simulink model "r_128w_512_11_r370"  (Simulink 7.2) (continued)
== system r_128w_512_11_r370 == (continued)
      mask help: Fold adders into DSPs: Causes adders to be absorbed into DSP blocks (supported in Virtex5)
      mask init: same as r_128w_512_11_r370/pfb_fir_real0
      settings: AttributesFormatString=taps=4, add_latency=1
      mask Help: eval('xlWeb(''http://casper.berkeley.edu/wiki/Pfb_fir_real'')')
      mask StyleString: edit,edit,popup(bartlett|barthannwin|blackman|blackmanharris|bohamwin|chebwin|flattopwin|gausswin|hamming|hann|kaiser|nuttallwin|parzenwin|rectwin|tukeywin|triang|userwindow),edit,edit,edit,edit,edit,edit,edit,edit,edit,edit,popup(Truncate|Round  (unbiased: +/- Inf)|Round  (unbiased: Even Values)),edit,checkbox,edit,checkbox
      mask CallbackString:
      | |%num_taps = eval(get_param(gcb, 'TotalTaps'));
      | %old_vec = eval(get_param(gcb, 'mult_spec'));=-0l
      | 
      | %vec = 2.*ones(1,num_taps);
      | %old_size = length(old_vec);
      | 
      | %if(old_size <= num_taps),
      | %    new_size = old_size;
      | %else,
      | %    new_size = num_taps;
      | %end
      | 
      | %for n = 1:new_size, vec(n) = old_vec(n); end
      | %set_param(gcb, 'mult_spec', mat2str(vec));||||||||||||||%thisVariable = 'specify_mult';
      | %affectedVars = {'mult_spec'};
      | 
      | %thisBlock = gcb();
      | %thisVarState = get_param(thisBlock, thisVariable);
      | %maskNames = get_param(thisBlock, 'MaskNames');
      | %maskEnables = get_param(thisBlock, 'MaskEnables');
      | 
      | %for varName = affectedVars
      | %    varIndex = find(ismember(maskNames, varName));
      | %    maskEnables{varIndex} = thisVarState;
      | %end
      | 
      | %set_param(thisBlock, 'MaskEnables', maskEnables);||
  pfb_fir_real2 : Subsystem  AncestorBlock=casper_library/PFBs/pfb_fir_real  mask(pfb_fir_real){PFBSize=10, TotalTaps=4, WindowType=hamming, n_inputs=2, MakeBiplex=0, BitWidthIn=8, BitWidthOut=18, CoeffBitWidth=12, CoeffDistMem=0, add_latency=1, mult_latency=2, bram_latency=2, conv_latency=2, quantization=Round  (unbiased: Even Values), fwidth=1, specify_mult=off, mult_spec=[2                                      2], adder_folding=on}
      mask prompts: PFBSize "Size of PFB: (2^? pnts)"; TotalTaps "Total Number of Taps:"; WindowType "Windowing Function:"; n_inputs "Number of Simultaneous Inputs: (2^?)"; MakeBiplex "Make Biplex"; BitWidthIn "Input Bitwidth:"; BitWidthOut "Output Bitwidth:"; CoeffBitWidth "Coefficient Bitwidth:"; CoeffDistMem "Use Distributed Memory for Coeffs"; add_latency "Add Latency"; mult_latency "Mult Latency"; bram_latency "BRAM Latency"; conv_latency "Convert latency"; quantization "Quantization Behavior"; fwidth "Bin Width Scaling (normal=1)"; specify_mult "Specify multiplier use (behavioral if not specified)"; mult_spec "Multiplier specification (0=core, 1=embedded, 2=behavioural) (left=1st tap)"; adder_folding "Fold adders into DSPs"
      mask help: Fold adders into DSPs: Causes adders to be absorbed into DSP blocks (supported in Virtex5)
      mask init: same as r_128w_512_11_r370/pfb_fir_real0
      settings: AttributesFormatString=taps=4, add_latency=1
      mask Help: eval('xlWeb(''http://casper.berkeley.edu/wiki/Pfb_fir_real'')')
      mask StyleString: edit,edit,popup(bartlett|barthannwin|blackman|blackmanharris|bohamwin|chebwin|flattopwin|gausswin|hamming|hann|kaiser|nuttallwin|parzenwin|rectwin|tukeywin|triang|userwindow),edit,edit,edit,edit,edit,edit,edit,edit,edit,edit,popup(Truncate|Round  (unbiased: +/- Inf)|Round  (unbiased: Even Values)),edit,checkbox,edit,checkbox
      mask CallbackString:
      | |%num_taps = eval(get_param(gcb, 'TotalTaps'));
      | %old_vec = eval(get_param(gcb, 'mult_spec'));=-0l
      | 
      | %vec = 2.*ones(1,num_taps);
      | %old_size = length(old_vec);
      | 
      | %if(old_size <= num_taps),
      | %    new_size = old_size;
      | %else,
      | %    new_size = num_taps;
      | %end
      | 
      | %for n = 1:new_size, vec(n) = old_vec(n); end
      | %set_param(gcb, 'mult_spec', mat2str(vec));||||||||||||||%thisVariable = 'specify_mult';
      | %affectedVars = {'mult_spec'};
      | 
      | %thisBlock = gcb();
      | %thisVarState = get_param(thisBlock, thisVariable);
      | %maskNames = get_param(thisBlock, 'MaskNames');
      | %maskEnables = get_param(thisBlock, 'MaskEnables');
      | 
      | %for varName = affectedVars
      | %    varIndex = find(ismember(maskNames, varName));
      | %    maskEnables{varIndex} = thisVarState;
      | %end
      | 
      | %set_param(thisBlock, 'MaskEnables', maskEnables);||
  pfb_fir_real3 : Subsystem  AncestorBlock=casper_library/PFBs/pfb_fir_real  mask(pfb_fir_real){PFBSize=10, TotalTaps=4, WindowType=hamming, n_inputs=2, MakeBiplex=0, BitWidthIn=8, BitWidthOut=18, CoeffBitWidth=12, CoeffDistMem=0, add_latency=1, mult_latency=2, bram_latency=2, conv_latency=2, quantization=Round  (unbiased: Even Values), fwidth=1, specify_mult=off, mult_spec=[2                                      2], adder_folding=on}
      mask prompts: PFBSize "Size of PFB: (2^? pnts)"; TotalTaps "Total Number of Taps:"; WindowType "Windowing Function:"; n_inputs "Number of Simultaneous Inputs: (2^?)"; MakeBiplex "Make Biplex"; BitWidthIn "Input Bitwidth:"; BitWidthOut "Output Bitwidth:"; CoeffBitWidth "Coefficient Bitwidth:"; CoeffDistMem "Use Distributed Memory for Coeffs"; add_latency "Add Latency"; mult_latency "Mult Latency"; bram_latency "BRAM Latency"; conv_latency "Convert latency"; quantization "Quantization Behavior"; fwidth "Bin Width Scaling (normal=1)"; specify_mult "Specify multiplier use (behavioral if not specified)"; mult_spec "Multiplier specification (0=core, 1=embedded, 2=behavioural) (left=1st tap)"; adder_folding "Fold adders into DSPs"
      mask help: Fold adders into DSPs: Causes adders to be absorbed into DSP blocks (supported in Virtex5)
      mask init: same as r_128w_512_11_r370/pfb_fir_real0
      settings: AttributesFormatString=taps=4, add_latency=1
      mask Help: eval('xlWeb(''http://casper.berkeley.edu/wiki/Pfb_fir_real'')')
      mask StyleString: edit,edit,popup(bartlett|barthannwin|blackman|blackmanharris|bohamwin|chebwin|flattopwin|gausswin|hamming|hann|kaiser|nuttallwin|parzenwin|rectwin|tukeywin|triang|userwindow),edit,edit,edit,edit,edit,edit,edit,edit,edit,edit,popup(Truncate|Round  (unbiased: +/- Inf)|Round  (unbiased: Even Values)),edit,checkbox,edit,checkbox
      mask CallbackString:
      | |%num_taps = eval(get_param(gcb, 'TotalTaps'));
      | %old_vec = eval(get_param(gcb, 'mult_spec'));=-0l
      | 
      | %vec = 2.*ones(1,num_taps);
      | %old_size = length(old_vec);
      | 
      | %if(old_size <= num_taps),
      | %    new_size = old_size;
      | %else,
      | %    new_size = num_taps;
      | %end
      | 
      | %for n = 1:new_size, vec(n) = old_vec(n); end
      | %set_param(gcb, 'mult_spec', mat2str(vec));||||||||||||||%thisVariable = 'specify_mult';
      | %affectedVars = {'mult_spec'};
      | 
      | %thisBlock = gcb();
      | %thisVarState = get_param(thisBlock, thisVariable);
      | %maskNames = get_param(thisBlock, 'MaskNames');
      | %maskEnables = get_param(thisBlock, 'MaskEnables');
      | 
      | %for varName = affectedVars
      | %    varIndex = find(ismember(maskNames, varName));
      | %    maskEnables{varIndex} = thisVarState;
      | %end
      | 
      | %set_param(thisBlock, 'MaskEnables', maskEnables);||
  pol_pack0 : Subsystem
  pol_pack1 : Subsystem
  pol_pack2 : Subsystem
  pol_pack3 : Subsystem
  posedge{1..2,21,23..24,30} (6 alike) : casper_library/Misc/posedge  Tag=Rising Edge Detector  SystemSampleTime=-1
  pps_count : xps_library/software register  Tag=xps:sw_reg  SystemSampleTime=-1  io_dir=To Processor  arith_type=Unsigned  bitwidth=32  bin_pt=0  sample_period=1  gw_name=r_128w_512_11_r370_pps_count_user_data_in
      link override convert: arith_type=Unsigned  n_bits=32  bin_pt=0
  quant0 : Subsystem  mask{channel_bits=9, din_bit_width=18, din_bin_pt=17, coeff_bit_width=16, coeff_bin_pt=1, dout_bit_width=4, dout_bin_pt=3, init_val=4, overflow=Saturate, quantization=Round  (unbiased: even values), conv_latency=2, add_latency=1, mult_latency=2, bram_latency=2}
      mask prompts: channel_bits "Number channels (2^?)"; din_bit_width "Data input bit width"; din_bin_pt "Data input binary point"; coeff_bit_width "Coefficient bit width"; coeff_bin_pt "Coefficient binary point"; dout_bit_width "Data out bit width"; dout_bin_pt "Data out binary point"; init_val "Initial value"; overflow "Overflow behaviour"; quantization "Quantization Behaviour"; conv_latency "Conversion latency"; add_latency "Adder latency"; mult_latency "Multiplier latency"; bram_latency "BRAM latency"
      mask init:
      | blk = gcb;
      | set_param([blk,'/convert_of0'], ...
      |     'bit_width_i', num2str(din_bit_width+coeff_bit_width+1), ...
      |     'binary_point_i', num2str(din_bin_pt+coeff_bin_pt), ...
      |     'bit_width_o', num2str(dout_bit_width), 'binary_point_o', num2str(dout_bin_pt), ...
      |     'latency', num2str(conv_latency), 'overflow', overflow, ...
      |     'quantization', quantization);
      | set_param([blk,'/convert_of1'], ...
      |     'bit_width_i', num2str(din_bit_width+coeff_bit_width+1), ...
      |     'binary_point_i', num2str(din_bin_pt+coeff_bin_pt), ...
      |     'bit_width_o', num2str(dout_bit_width), 'binary_point_o', num2str(dout_bin_pt), ...
      |     'latency', num2str(conv_latency), 'overflow', overflow, ...
      |     'quantization', quantization);
      | set_param([blk,'/convert_of2'], ...
      |     'bit_width_i', num2str(din_bit_width+coeff_bit_width+1), ...
      |     'binary_point_i', num2str(din_bin_pt+coeff_bin_pt), ...
      |     'bit_width_o', num2str(dout_bit_width), 'binary_point_o', num2str(dout_bin_pt), ...
      |     'latency', num2str(conv_latency), 'overflow', overflow, ...
      |     'quantization', quantization);
      | set_param([blk,'/convert_of3'], ...
      |     'bit_width_i', num2str(din_bit_width+coeff_bit_width+1), ...
      |     'binary_point_i', num2str(din_bin_pt+coeff_bin_pt), ...
      |     'bit_width_o', num2str(dout_bit_width), 'binary_point_o', num2str(dout_bin_pt), ...
      |     'latency', num2str(conv_latency), 'overflow', overflow, ...
      |     'quantization', quantization);
      mask StyleString: edit,edit,edit,edit,edit,edit,edit,edit,popup(Wrap|Saturate|Error),popup(Truncate|Round  (unbiased: +/- Inf)|Round  (unbiased: even values)),edit,edit,edit,edit
  quant1 : Subsystem  mask{channel_bits=9, din_bit_width=18, din_bin_pt=17, coeff_bit_width=16, coeff_bin_pt=1, dout_bit_width=4, dout_bin_pt=3, init_val=4, overflow=Saturate, quantization=Round  (unbiased: even values), conv_latency=2, add_latency=1, mult_latency=2, bram_latency=2}
      mask prompts: channel_bits "Number channels (2^?)"; din_bit_width "Data input bit width"; din_bin_pt "Data input binary point"; coeff_bit_width "Coefficient bit width"; coeff_bin_pt "Coefficient binary point"; dout_bit_width "Data out bit width"; dout_bin_pt "Data out binary point"; init_val "Initial value"; overflow "Overflow behaviour"; quantization "Quantization Behaviour"; conv_latency "Conversion latency"; add_latency "Adder latency"; mult_latency "Multiplier latency"; bram_latency "BRAM latency"
      mask init: same as r_128w_512_11_r370/quant0
      mask StyleString: edit,edit,edit,edit,edit,edit,edit,edit,popup(Wrap|Saturate|Error),popup(Truncate|Round  (unbiased: +/- Inf)|Round  (unbiased: even values)),edit,edit,edit,edit
  quant2 : Subsystem  mask{channel_bits=9, din_bit_width=18, din_bin_pt=17, coeff_bit_width=16, coeff_bin_pt=1, dout_bit_width=4, dout_bin_pt=3, init_val=4, overflow=Saturate, quantization=Round  (unbiased: even values), conv_latency=2, add_latency=1, mult_latency=2, bram_latency=2}
      mask prompts: channel_bits "Number channels (2^?)"; din_bit_width "Data input bit width"; din_bin_pt "Data input binary point"; coeff_bit_width "Coefficient bit width"; coeff_bin_pt "Coefficient binary point"; dout_bit_width "Data out bit width"; dout_bin_pt "Data out binary point"; init_val "Initial value"; overflow "Overflow behaviour"; quantization "Quantization Behaviour"; conv_latency "Conversion latency"; add_latency "Adder latency"; mult_latency "Multiplier latency"; bram_latency "BRAM latency"
      mask init: same as r_128w_512_11_r370/quant0
      mask StyleString: edit,edit,edit,edit,edit,edit,edit,edit,popup(Wrap|Saturate|Error),popup(Truncate|Round  (unbiased: +/- Inf)|Round  (unbiased: even values)),edit,edit,edit,edit
  quant3 : Subsystem  mask{channel_bits=9, din_bit_width=18, din_bin_pt=17, coeff_bit_width=16, coeff_bin_pt=1, dout_bit_width=4, dout_bin_pt=3, init_val=4, overflow=Saturate, quantization=Round  (unbiased: even values), conv_latency=2, add_latency=1, mult_latency=2, bram_latency=2}
      mask prompts: channel_bits "Number channels (2^?)"; din_bit_width "Data input bit width"; din_bin_pt "Data input binary point"; coeff_bit_width "Coefficient bit width"; coeff_bin_pt "Coefficient binary point"; dout_bit_width "Data out bit width"; dout_bin_pt "Data out binary point"; init_val "Initial value"; overflow "Overflow behaviour"; quantization "Quantization Behaviour"; conv_latency "Conversion latency"; add_latency "Adder latency"; mult_latency "Multiplier latency"; bram_latency "BRAM latency"
      mask init: same as r_128w_512_11_r370/quant0
      mask StyleString: edit,edit,edit,edit,edit,edit,edit,edit,popup(Wrap|Saturate|Error),popup(Truncate|Round  (unbiased: +/- Inf)|Round  (unbiased: even values)),edit,edit,edit,edit
  quant_snap0 : casper_library/Scopes/snap  SystemSampleTime=-1  nsamples=10
  repack0 : Subsystem
  revision_control : Subsystem  AncestorBlock=casper_library/Misc/revision_control  mask(revision_control){rev_major=0, rev_minor=370, error_on_dirty=off, error_on_norcs=off}
      mask prompts: rev_major "Major revision number"; rev_minor "Minor revision number"; error_on_dirty "Error on out-of-date"; error_on_norcs "Error on no revision control"
      mask init:
      | revision_control_init( gcb, ...
      |   'rev_major', rev_major, ...
      |   'rev_minor', rev_minor, ...
      |   'error_on_dirty', error_on_dirty, ...
      |   'error_on_norcs', error_on_norcs);
  timing : Subsystem  mask{reset_period=1, sync_gen_bits=27}
      mask prompts: reset_period "Master reset time (sync pulses)"; sync_gen_bits "Size of sync generator counter (bits)"
  unpack0 : Subsystem
  unpack1 : Subsystem
  unpack2 : Subsystem
  unpack3 : Subsystem
  wires:
  [adc_sync0] -> Logical3/2
  [adc0] -> Concat/1
  [adc1] -> Concat/2
  timing/2(sync) -> [sync]
  timing/3(data_out) -> adc_unpack/1(In1)
  adc_unpack/2(Out2) -> [data_adc1]
  Logical3/1 -> timing/4(pps), [pps]
  Concat/1 -> timing/2(data_in)
  Constant9/1 -> fft_shift0/1
  fft_shift0/1 -> Slice6/1
  a0p0_1st_shift/2(dout1) -> Gateway Out1/1, fft_wideband_real0/3(in0)
  a0p0_1st_shift/3(dout2) -> Gateway Out2/1, fft_wideband_real0/4(in1)
  a0p0_1st_shift/4(dout3) -> Gateway Out3/1, fft_wideband_real0/5(in2)
  a0p0_1st_shift/5(dout4) -> Gateway Out4/1, fft_wideband_real0/6(in3)
  Slice6/1 -> Gateway Out5/1, fft_wideband_real0/2(shift)
  a0p0_1st_shift/1(sync_out) -> Gateway Out/1, fft_wideband_real0/1(sync)
  fft_wideband_real0/1(sync_out) -> Gateway Out6/1, [fft_sync], fd_fs0/1(sync)
  fft_wideband_real0/2(out0) -> c_to_ri6/1, fd_fs0/8(din0)
  fft_wideband_real0/3(out1) -> c_to_ri7/1, fd_fs0/9(din1)
  fft_wideband_real0/4(of) -> [fft_or0]
  Gateway Out/1 -> Scope/1
  Gateway Out1/1 -> Scope/2
  Gateway Out2/1 -> Scope/3
  Gateway Out3/1 -> Scope/4
  Gateway Out4/1 -> Scope/5
  Gateway Out5/1 -> Scope/6
  adc_unpack/1(Out1) -> [data_adc0]
  [adc2] -> Concat/3
  [adc3] -> Concat/4
  adc_unpack/3(Out3) -> [data_adc2]
  adc_unpack/4(Out4) -> [data_adc3]
  pfb_fir_real1/2(pol1_out1) -> a0p1_1st_shift/2(din1)
  pfb_fir_real1/3(pol1_out2) -> a0p1_1st_shift/3(din2)
  pfb_fir_real1/4(pol1_out3) -> a0p1_1st_shift/4(din3)
  pfb_fir_real1/5(pol1_out4) -> a0p1_1st_shift/5(din4)
  a0p1_1st_shift/2(dout1) -> fft_wideband_real1/3(in0)
  a0p1_1st_shift/3(dout2) -> fft_wideband_real1/4(in1)
  a0p1_1st_shift/4(dout3) -> fft_wideband_real1/5(in2)
  a0p1_1st_shift/5(dout4) -> fft_wideband_real1/6(in3)
  pfb_fir_real1/1(sync_out) -> a0p1_1st_shift/1(sync_in)
  a0p1_1st_shift/1(sync_out) -> fft_wideband_real1/1(sync)
  fft_wideband_real1/4(of) -> [fft_or1]
  delay_wideband_prog2/1(sync_out) -> pfb_fir_real2/1(sync)
  delay_wideband_prog2/2(data_out1) -> pfb_fir_real2/2(pol1_in1)
  delay_wideband_prog2/3(data_out2) -> pfb_fir_real2/3(pol1_in2)
  delay_wideband_prog2/4(data_out3) -> pfb_fir_real2/4(pol1_in3)
  delay_wideband_prog2/5(data_out4) -> pfb_fir_real2/5(pol1_in4)
  pfb_fir_real2/2(pol1_out1) -> a1p0_1st_shift/2(din1)
  pfb_fir_real2/3(pol1_out2) -> a1p0_1st_shift/3(din2)
  pfb_fir_real2/4(pol1_out3) -> a1p0_1st_shift/4(din3)
  pfb_fir_real2/5(pol1_out4) -> a1p0_1st_shift/5(din4)
  a1p0_1st_shift/2(dout1) -> fft_wideband_real2/3(in0)
  a1p0_1st_shift/3(dout2) -> fft_wideband_real2/4(in1)
  a1p0_1st_shift/4(dout3) -> fft_wideband_real2/5(in2)
  a1p0_1st_shift/5(dout4) -> fft_wideband_real2/6(in3)
  pfb_fir_real2/1(sync_out) -> a1p0_1st_shift/1(sync_in)
  a1p0_1st_shift/1(sync_out) -> fft_wideband_real2/1(sync)
  fft_wideband_real2/4(of) -> [fft_or2]
  Concat5/1 -> [quant2_data]
  delay_wideband_prog3/1(sync_out) -> pfb_fir_real3/1(sync)
  delay_wideband_prog3/2(data_out1) -> pfb_fir_real3/2(pol1_in1)
  delay_wideband_prog3/3(data_out2) -> pfb_fir_real3/3(pol1_in2)
  delay_wideband_prog3/4(data_out3) -> pfb_fir_real3/4(pol1_in3)
  delay_wideband_prog3/5(data_out4) -> pfb_fir_real3/5(pol1_in4)
  pfb_fir_real3/2(pol1_out1) -> a1p1_1st_shift/2(din1)
  pfb_fir_real3/3(pol1_out2) -> a1p1_1st_shift/3(din2)
  pfb_fir_real3/4(pol1_out3) -> a1p1_1st_shift/4(din3)
  pfb_fir_real3/5(pol1_out4) -> a1p1_1st_shift/5(din4)
  a1p1_1st_shift/2(dout1) -> fft_wideband_real3/3(in0)
  a1p1_1st_shift/3(dout2) -> fft_wideband_real3/4(in1)
  a1p1_1st_shift/4(dout3) -> fft_wideband_real3/5(in2)
  a1p1_1st_shift/5(dout4) -> fft_wideband_real3/6(in3)
  pfb_fir_real3/1(sync_out) -> a1p1_1st_shift/1(sync_in)
  a1p1_1st_shift/1(sync_out) -> fft_wideband_real3/1(sync)
  fft_wideband_real3/4(of) -> [fft_or3]
  quant3/2(dout0) -> Subsystem1/5(In5), Concat8/1
  quant3/3(dout1) -> Concat8/2
  Concat8/1 -> [quant3_data]
  quant3/4(of) -> Subsystem1/9(In9), [quant_or3]
  Gateway Out6/1 -> Scope2/1
  Gateway Out7/1 -> Scope2/2
  Gateway Out8/1 -> Scope2/3
  Gateway Out9/1 -> Scope2/4
  Gateway Out10/1 -> Scope2/5
  c_to_ri6/1 -> Gateway Out7/1
  c_to_ri6/2 -> Gateway Out8/1
  c_to_ri7/1 -> Gateway Out9/1
  c_to_ri7/2 -> Gateway Out10/1
  unpack0/1(Out1) -> Gateway Out12/1, delay_wideband_prog0/4(data_in1)
  unpack0/2(Out2) -> Gateway Out13/1, delay_wideband_prog0/5(data_in2)
  unpack0/3(Out3) -> Gateway Out14/1, delay_wideband_prog0/6(data_in3)
  unpack0/4(Out4) -> Gateway Out15/1, delay_wideband_prog0/7(data_in4)
  delay_wideband_prog1/1(sync_out) -> pfb_fir_real1/1(sync)
  delay_wideband_prog1/2(data_out1) -> pfb_fir_real1/2(pol1_in1)
  delay_wideband_prog1/3(data_out2) -> pfb_fir_real1/3(pol1_in2)
  delay_wideband_prog1/4(data_out3) -> pfb_fir_real1/4(pol1_in3)
  delay_wideband_prog1/5(data_out4) -> pfb_fir_real1/5(pol1_in4)
  delay_wideband_prog0/1(sync_out) -> Gateway Out17/1, pfb_fir_real0/1(sync)
  delay_wideband_prog0/2(data_out1) -> Gateway Out18/1, pfb_fir_real0/2(pol1_in1)
  delay_wideband_prog0/3(data_out2) -> Gateway Out19/1, pfb_fir_real0/3(pol1_in2)
  delay_wideband_prog0/4(data_out3) -> Gateway Out20/1, pfb_fir_real0/4(pol1_in3)
  delay_wideband_prog0/5(data_out4) -> Gateway Out21/1, pfb_fir_real0/5(pol1_in4)
  Gateway Out11/1 -> Scope3/1
  Gateway Out12/1 -> Scope3/2
  Gateway Out13/1 -> Scope3/3
  Gateway Out14/1 -> Scope3/4
  Gateway Out15/1 -> Scope3/5
  Gateway Out16/1 -> Scope3/6
  Gateway Out17/1 -> Scope4/1
  Gateway Out18/1 -> Scope4/2
  Gateway Out19/1 -> Scope4/3
  Gateway Out20/1 -> Scope4/4
  Gateway Out21/1 -> Scope4/5
  Gateway Out22/1 -> Scope5/1
  Gateway Out23/1 -> Scope5/2
  Gateway Out24/1 -> Scope5/3
  Gateway Out25/1 -> Scope5/4
  Gateway Out26/1 -> Scope5/5
  [adc_sync1] -> Logical3/3
  quant2/2(dout0) -> Subsystem1/4(In4), Concat5/1
  quant2/3(dout1) -> Concat5/2
  quant2/4(of) -> Subsystem1/8(In8), [quant_or2]
  quant1/2(dout0) -> Subsystem1/3(In3), Concat3/1
  quant1/3(dout1) -> Concat3/2
  Concat3/1 -> [quant1_data]
  quant1/4(of) -> Subsystem1/7(In7), [quant_or1]
  quant0/2(dout0) -> Concat7/1, Subsystem1/2(In2)
  quant0/3(dout1) -> Concat7/2
  quant0/1(sync_out) -> Subsystem1/1(In1), [quant0_sync]
  quant0/4(of) -> [quant_or0], Subsystem1/6(In6)
  Concat7/1 -> [quant0_data]
  timing/1(armed) -> Logical14/2, [armed]
  Logical14/1 -> [mrst]
  [mrst] -> cd_local_time/1(reset)
  [sync] -> cd_local_time/2(sync)
  cd_local_time/1(time) -> [cd_local_time]
  [sys_rst] -> Logical14/1
  [man_sync] -> Logical3/1
  [arm] -> timing/1(arm)
  Constant2/1 -> timing/3(sync_period)
  pfb_fir_real0/2(pol1_out1) -> a0p0_1st_shift/2(din1), Gateway Out23/1
  pfb_fir_real0/3(pol1_out2) -> a0p0_1st_shift/3(din2), Gateway Out24/1
  pfb_fir_real0/4(pol1_out3) -> a0p0_1st_shift/4(din3), Gateway Out25/1
  pfb_fir_real0/5(pol1_out4) -> a0p0_1st_shift/5(din4), Gateway Out26/1
  pfb_fir_real0/1(sync_out) -> a0p0_1st_shift/1(sync_in), Gateway Out22/1
  quant1/1(sync_out) -> Terminator6/1
  quant2/1(sync_out) -> Terminator7/1
  quant3/1(sync_out) -> Terminator8/1
  Constant33/1 -> ld_time_msw0/1
  Constant29/1 -> ld_time_lsw0/1
  ld_time_msw0/1 -> Slice73/1, Slice7/1
  Slice73/1 -> Concat9/1
  ld_time_lsw0/1 -> Concat9/2
  fstatus0/1 -> Terminator9/1
  Constant18/1 -> control/1
  control/1 -> Delay6/1
  Subsystem/1(status) -> fstatus0/1
  Delay6/1 -> Slice78/1, man_sync1/1, arm1/1, arm2/1, arm3/1, arm4/1, arm5/1, arm6/1, arm7/1
  Slice78/1 -> [sys_rst]
  man_sync1/1 -> posedge1/1
  posedge1/1 -> [man_sync]
  arm1/1 -> posedge24/1
  posedge24/1 -> [arm]
  arm2/1 -> posedge23/1
  posedge23/1 -> [clr_status]
  Counter1/1 -> Register19/1
  Register19/1 -> clk_frequency/1
  [pps] -> Counter1/1, Counter3/2, Register19/2
  clk_frequency/1 -> Terminator10/1
  pps_count/1 -> Terminator11/1
  [mrst] -> Counter3/1
  [cd_local_time] -> Delay7/1
  mcount_msw/1 -> Terminator12/1
  mcount_lsw/1 -> Terminator13/1
  Slice/1 -> mcount_lsw/1
  Delay7/1 -> Slice1/1, Slice/1
  Slice1/1 -> mcount_msw/1
  Constant1/1 -> adc_snap0/3
  [sync] -> adc_snap0/2
  Constant6/1 -> adc_snap1/3
  [data_adc1] -> adc_snap1/1
  [sync] -> adc_snap1/2
  Constant7/1 -> adc_snap2/3
  [data_adc2] -> adc_snap2/1
  [sync] -> adc_snap2/2
  [data_adc0] -> adc_snap0/1
  Constant19/1 -> adc_snap3/3
  [sync] -> adc_snap3/2
  [data_adc3] -> adc_snap3/1
  Logical7/1 -> Logical11/1
  Logical10/1 -> Logical11/2
  [sync] -> Counter/2
  [mrst] -> Counter/1
  Logical11/1 -> leds/1(warning)
  Counter/1 -> leds/2(sync_led)
  [pps] -> Counter2/1
  Counter2/1 -> leds/3(pps)
  [adc_or0] -> Logical7/1
  [fft_or0] -> Logical7/2
  [adc_or1] -> Logical7/3
  [fft_or1] -> Logical7/4
  [adc_or0] -> Logical10/1
  [fft_or2] -> Logical10/2
  [adc_or1] -> Logical10/3
  [fft_or3] -> Logical10/4
  fstatus1/1 -> Terminator2/1
  fstatus2/1 -> Terminator3/1
  fstatus3/1 -> Terminator4/1
  Constant32/1 -> coarse_delay0/1
  Constant8/1 -> a0_fd0/1
  Constant4/1 -> a1_fd0/1
  a0_fd0/1 -> Slice3/1, Slice2/1
  a1_fd0/1 -> Slice5/1, Slice4/1
  Slice4/1 -> Concat22/4
  Slice5/1 -> Concat22/5
  Concat9/1 -> cd_tr0/4(load_time), fd_tr0/4(load_time)
  [cd_local_time] -> cd_tr0/3(current_time)
  Slice7/1 -> fd_tr0/2(arm), cd_tr0/2(arm)
  [mrst] -> cd_tr0/1(rst)
  delay_tr_status0/1 -> Terminator14/1
  cd_tr0/1(arm_count) -> Convert/1
  cd_tr0/4(ld_count) -> Convert1/1
  cd_tr0/3(enable) -> [cd_en0]
  cd_tr0/2(value) -> [cd_val0]
  Convert/1 -> Concat2/1
  Convert1/1 -> Concat2/2
  Concat2/1 -> delay_tr_status0/1
  fd_tr0/2(value) -> [fd_val0]
  fd_tr0/3(enable) -> [fd_en0]
  delay_tr_status1/1 -> Terminator16/1
  cd_tr1/1(arm_count) -> Convert4/1
  cd_tr1/2(value) -> [cd_val1]
  Convert4/1 -> Concat10/1
  Convert5/1 -> Concat10/2
  Concat10/1 -> delay_tr_status1/1
  fd_tr1/2(value) -> [fd_val1]
  [cd_local_time] -> cd_tr2/3(current_time)
  [mrst] -> fd_tr2/1(rst)
  fd_fs0/3(dout1) -> quant0/7(din1)
  Constant27/1 -> adc_ctrl0/1
  adc_ctrl0/1 -> Slice29/1, Slice30/1
  [eq_real0] -> quant0/1(real_in0)
  Logical/1 -> posedge21/1
  Logical6/1 -> posedge30/1
  posedge21/1 -> [adc_sync0]
  posedge30/1 -> [adc_sync1]
  [mrst] -> fd_local_time/1(reset)
  [fft_sync] -> fd_local_time/2(sync)
  fd_local_time/1(time) -> [fd_local_time]
  Constant43/1 -> XAUI0/1
  Constant44/1 -> XAUI0/2
  packetiser0/1(data_out) -> XAUI0/3
  packetiser0/2(oob) -> XAUI0/4
  packetiser0/3(valid_out) -> XAUI0/5
  XAUI0/2 -> Terminator27/1
  XAUI0/3 -> Terminator32/1
  XAUI0/6 -> [xaui_of0]
  Logical4/1 -> neg_edge_ext4/1(in)
  XAUI0/4 -> Terminator33/1
  XAUI0/1 -> Terminator26/1
  neg_edge_ext4/1(out) -> leds/4(comms)
  [xaui_of0] -> Logical4/1
  [xaui_ldn0] -> Logical4/2
  [xaui_of1] -> Logical4/3
  [xaui_ldn1] -> Logical4/4
  Subsystem2/1(status) -> fstatus1/1
  Subsystem3/1(status) -> fstatus2/1
  Subsystem4/1(status) -> fstatus3/1
  [armed] -> Concat21/1
  Counter3/1 -> Concat21/2
  Concat21/1 -> pps_count/1
  Constant40/1 -> fft_shift1/1
  fft_shift1/1 -> Slice26/1
  Slice26/1 -> fft_wideband_real1/2(shift)
  Constant42/1 -> fft_shift2/1
  fft_shift2/1 -> Slice27/1
  Slice27/1 -> fft_wideband_real2/2(shift)
  Constant45/1 -> fft_shift3/1
  fft_shift3/1 -> Slice28/1
  Slice28/1 -> fft_wideband_real3/2(shift)
  XAUI0/5 -> [xaui_ldn0]
  calc_adc_sum_sq/1(sum_sq) -> adc_sum_sq0/1
  decat0/1(Out1) -> calc_adc_sum_sq/1(In1)
  decat0/2(Out2) -> calc_adc_sum_sq/2(In2)
  decat0/3(Out3) -> calc_adc_sum_sq/3(In3)
  decat0/4(Out4) -> calc_adc_sum_sq/4(In4)
  [adc0] -> decat0/1(In1)
  adc_sum_sq0/1 -> Terminator/1
  [adc1] -> decat1/1(In1)
  [adc2] -> decat2/1(In1)
  [adc3] -> decat3/1(In1)
  katadc0/15(data_valid) -> Terminator1/1
  katadc0/1(i0) -> pol_pack0/1(In1)
  katadc0/2(i1) -> pol_pack0/2(In2)
  katadc0/3(i2) -> pol_pack0/3(In3)
  katadc0/4(i3) -> pol_pack0/4(In4)
  katadc0/5(q0) -> pol_pack1/1(In1)
  katadc0/6(q1) -> pol_pack1/2(In2)
  katadc0/7(q2) -> pol_pack1/3(In3)
  katadc0/8(q3) -> pol_pack1/4(In4)
  katadc0/11(sync0) -> Logical/1
  katadc0/12(sync1) -> Logical/2
  katadc0/13(sync2) -> Logical/3
  katadc0/14(sync3) -> Logical/4
  katadc1/1(i0) -> pol_pack2/1(In1)
  katadc1/2(i1) -> pol_pack2/2(In2)
  katadc1/3(i2) -> pol_pack2/3(In3)
  katadc1/4(i3) -> pol_pack2/4(In4)
  katadc1/5(q0) -> pol_pack3/1(In1)
  katadc1/6(q1) -> pol_pack3/2(In2)
  katadc1/7(q2) -> pol_pack3/3(In3)
  katadc1/8(q3) -> pol_pack3/4(In4)
  katadc1/11(sync0) -> Logical6/1
  katadc1/12(sync1) -> Logical6/2
  katadc1/13(sync2) -> Logical6/3
  katadc1/14(sync3) -> Logical6/4
  katadc1/15(data_valid) -> Terminator5/1
  Constant/1 -> katadc0/8(sim_data_valid)
  Pulse Generator/1 -> katadc0/7(sim_sync)
  Pulse Generator2/1 -> katadc0/6(sim_q)
  Pulse Generator1/1 -> katadc0/3(sim_i)
  Slice29/1 -> katadc0/1(en_i)
  Slice30/1 -> katadc0/2(atten_i)
  Constant48/1 -> adc_ctrl1/1
  adc_ctrl1/1 -> Slice34/1, Slice33/1
  Slice33/1 -> katadc0/4(en_q)
  Slice34/1 -> katadc0/5(atten_q)
  Constant49/1 -> adc_ctrl2/1
  adc_ctrl2/1 -> Slice32/1, Slice31/1
  Constant5/1 -> katadc1/8(sim_data_valid)
  Pulse Generator3/1 -> katadc1/7(sim_sync)
  Pulse Generator5/1 -> katadc1/6(sim_q)
  Pulse Generator4/1 -> katadc1/3(sim_i)
  Slice31/1 -> katadc1/1(en_i)
  Slice32/1 -> katadc1/2(atten_i)
  Constant50/1 -> adc_ctrl3/1
  adc_ctrl3/1 -> Slice35/1, Slice36/1
  Slice35/1 -> katadc1/4(en_q)
  Slice36/1 -> katadc1/5(atten_q)
  calc_adc_sum_sq1/1(sum_sq) -> adc_sum_sq1/1
  decat1/1(Out1) -> calc_adc_sum_sq1/1(In1)
  decat1/2(Out2) -> calc_adc_sum_sq1/2(In2)
  decat1/3(Out3) -> calc_adc_sum_sq1/3(In3)
  decat1/4(Out4) -> calc_adc_sum_sq1/4(In4)
  adc_sum_sq1/1 -> Terminator25/1
  calc_adc_sum_sq2/1(sum_sq) -> adc_sum_sq2/1
  decat2/1(Out1) -> calc_adc_sum_sq2/1(In1)
  decat2/2(Out2) -> calc_adc_sum_sq2/2(In2)
  decat2/3(Out3) -> calc_adc_sum_sq2/3(In3)
  decat2/4(Out4) -> calc_adc_sum_sq2/4(In4)
  adc_sum_sq2/1 -> Terminator30/1
  calc_adc_sum_sq3/1(sum_sq) -> adc_sum_sq3/1
  decat3/1(Out1) -> calc_adc_sum_sq3/1(In1)
  decat3/2(Out2) -> calc_adc_sum_sq3/2(In2)
  decat3/3(Out3) -> calc_adc_sum_sq3/3(In3)
  decat3/4(Out4) -> calc_adc_sum_sq3/4(In4)
  adc_sum_sq3/1 -> Terminator31/1
  Constant3/1 -> board_id/1
  board_id/1 -> [board_id]
  arm3/1 -> posedge2/1
  posedge2/1 -> [tvg_en]
  arm4/1 -> [ct_tvg]
  arm5/1 -> [packetiser_tvg]
  arm6/1 -> [fd_fs_tvg]
  repack0/1(dout0) -> quant_snap0/1
  repack0/2(sync_out) -> quant_snap0/2, Counter4/1
  [quant0_sync] -> repack0/1(sync)
  [quant0_data] -> repack0/2(din0)
  Counter4/1 -> Slice37/1
  Slice37/1 -> quant_snap0/3
  [clr_status] -> Subsystem/1(clr)
  [xaui_ldn0] -> Subsystem/2(xuai_ldn)
  [xaui_of0] -> Subsystem/3(xaui_of)
  [ct_err0] -> Subsystem/4(ct_err)
  [adc_or0] -> Subsystem/5(adc_or)
  [fft_or0] -> Subsystem/6(fft_or)
  [quant_or0] -> Subsystem/7(quant_or)
  [clr_status] -> Subsystem2/1(clr)
  [xaui_ldn0] -> Subsystem2/2(xuai_ldn)
  [xaui_of0] -> Subsystem2/3(xaui_of)
  [ct_err0] -> Subsystem2/4(ct_err)
  [adc_or0] -> Subsystem2/5(adc_or)
  [fft_or1] -> Subsystem2/6(fft_or)
  [quant_or1] -> Subsystem2/7(quant_or)
  [clr_status] -> Subsystem3/1(clr)
  [xaui_ldn1] -> Subsystem3/2(xuai_ldn)
  [xaui_of1] -> Subsystem3/3(xaui_of)
  [ct_err1] -> Subsystem3/4(ct_err)
  [adc_or1] -> Subsystem3/5(adc_or)
  [fft_or2] -> Subsystem3/6(fft_or)
  [quant_or2] -> Subsystem3/7(quant_or)
  [clr_status] -> Subsystem4/1(clr)
  [xaui_ldn1] -> Subsystem4/2(xuai_ldn)
  [xaui_of1] -> Subsystem4/3(xaui_of)
  [ct_err1] -> Subsystem4/4(ct_err)
  [adc_or1] -> Subsystem4/5(adc_or)
  [fft_or3] -> Subsystem4/6(fft_or)
  [quant_or3] -> Subsystem4/7(quant_or)
  katadc0/9(outofrange0) -> Logical1/1
  katadc0/10(outofrange1) -> Logical1/2
  Logical1/1 -> [adc_or0]
  Logical2/1 -> [adc_or1]
  katadc1/9(outofrange0) -> Logical2/1
  katadc1/10(outofrange1) -> Logical2/2
  [mrst] -> packetiser0/4(mrst_in)
  [board_id] -> packetiser0/5(ant_base)
  [quant0_sync] -> ct0/1(sync)
  [quant0_data] -> ct0/2(p0)
  [quant1_data] -> ct0/3(p1)
  ct0/4(ct_err) -> [ct_err0]
  [quant2_data] -> ct1/2(p0)
  fd_fs0/1(sync_out) -> [fd_sync], quant0/5(sync_in)
  [fd_sync] -> Counter8/1
  Counter8/1 -> eq0/1(addr)
  eq0/1(data_out) -> Slice41/1, Slice42/1
  Slice41/1 -> c_to_ri/1
  Slice42/1 -> c_to_ri1/1
  Constant41/1 -> eq0/2(data_in)
  Constant46/1 -> eq0/3(we)
  c_to_ri/1 -> [eq_real0]
  c_to_ri/2 -> [eq_imag0]
  c_to_ri1/1 -> [eq_real1]
  c_to_ri1/2 -> [eq_imag1]
  [fd_sync] -> Counter9/1
  Counter9/1 -> eq1/1(addr)
  eq1/1(data_out) -> Slice44/1, Slice43/1
  Slice43/1 -> c_to_ri2/1
  Slice44/1 -> c_to_ri3/1
  Constant28/1 -> eq1/2(data_in)
  Constant30/1 -> eq1/3(we)
  c_to_ri2/1 -> [eq_real2]
  c_to_ri2/2 -> [eq_imag2]
  c_to_ri3/1 -> [eq_real3]
  c_to_ri3/2 -> [eq_imag3]
  [fd_sync] -> Counter10/1
  Counter10/1 -> eq2/1(addr)
  eq2/1(data_out) -> Slice46/1, Slice45/1
  Slice45/1 -> c_to_ri4/1
  Slice46/1 -> c_to_ri5/1
  Constant35/1 -> eq2/2(data_in)
  Constant36/1 -> eq2/3(we)
  c_to_ri4/1 -> [eq_real4]
  c_to_ri4/2 -> [eq_imag4]
  c_to_ri5/1 -> [eq_real5]
  c_to_ri5/2 -> [eq_imag5]
  [fd_sync] -> Counter11/1
  Counter11/1 -> eq3/1(addr)
  eq3/1(data_out) -> Slice47/1, Slice48/1
  Slice47/1 -> c_to_ri8/1
  Slice48/1 -> c_to_ri9/1
  Constant31/1 -> eq3/2(data_in)
  Constant34/1 -> eq3/3(we)
  c_to_ri8/1 -> [eq_real6]
  c_to_ri8/2 -> [eq_imag6]
  c_to_ri9/1 -> [eq_real7]
  c_to_ri9/2 -> [eq_imag7]
  [eq_imag0] -> quant0/2(imag_in0)
  [eq_real1] -> quant0/3(real_in1)
  [eq_imag1] -> quant0/4(real_in2)
  [eq_real2] -> quant1/1(real_in0)
  [eq_imag2] -> quant1/2(imag_in0)
  [eq_real3] -> quant1/3(real_in1)
  [eq_imag3] -> quant1/4(real_in2)
  [eq_real4] -> quant2/1(real_in0)
  [eq_imag4] -> quant2/2(imag_in0)
  [eq_real5] -> quant2/3(real_in1)
  [eq_imag5] -> quant2/4(real_in2)
  fd_fs1/1(sync_out) -> quant1/5(sync_in)
  fd_fs1/2(dout0) -> quant1/6(din0)
  fd_fs1/3(dout1) -> quant1/7(din1)
  fd_fs2/1(sync_out) -> quant2/5(sync_in)
  fd_fs2/2(dout0) -> quant2/6(din0)
  fd_fs2/3(dout1) -> quant2/7(din1)
  [eq_real6] -> quant3/1(real_in0)
  [eq_imag6] -> quant3/2(imag_in0)
  [eq_real7] -> quant3/3(real_in1)
  [eq_imag7] -> quant3/4(real_in2)
  fd_fs3/1(sync_out) -> quant3/5(sync_in)
  fd_fs3/2(dout0) -> quant3/6(din0)
  fd_fs3/3(dout1) -> quant3/7(din1)
  XAUI0/7 -> Terminator34/1
  Constant37/1 -> XAUI1/1
  Constant38/1 -> XAUI1/2
  packetiser1/1(data_out) -> XAUI1/3
  packetiser1/2(oob) -> XAUI1/4
  packetiser1/3(valid_out) -> XAUI1/5
  XAUI1/2 -> Terminator23/1
  XAUI1/3 -> Terminator24/1
  XAUI1/6 -> [xaui_of1]
  XAUI1/4 -> Terminator28/1
  XAUI1/1 -> Terminator22/1
  XAUI1/5 -> [xaui_ldn1]
  [mrst] -> packetiser1/4(mrst_in)
  [board_id] -> packetiser1/5(ant_base)
  ct1/4(ct_err) -> [ct_err1]
  XAUI1/7 -> Terminator29/1
  [quant0_sync] -> ct1/1(sync)
  [quant3_data] -> ct1/3(p1)
  fd_fs0/2(dout0) -> quant0/6(din0)
  arm7/1 -> [adc_tvg]
  [cd_val0] -> Slice50/1, Slice53/1, Slice52/1, Slice49/1, Slice51/1
  ct0/1(sync_out) -> packetiser0/1(sync_in)
  ct0/2(data_valid) -> packetiser0/2(data_valid)
  ct0/3(data_out) -> packetiser0/3(data_in)
  ct1/1(sync_out) -> packetiser1/1(sync_in)
  ct1/2(data_valid) -> packetiser1/2(data_valid)
  ct1/3(data_out) -> packetiser1/3(data_in)
  [mrst] -> fd_tr0/1(rst)
  fd_tr0/1(arm_count) -> Terminator15/1
  fd_tr0/4(ld_count) -> Terminator51/1
  [mrst] -> cd_tr1/1(rst)
  [cd_local_time] -> cd_tr1/3(current_time)
  [fd_local_time] -> fd_tr0/3(current_time)
  [mrst] -> fd_tr1/1(rst)
  [fd_local_time] -> fd_tr1/3(current_time)
  fd_tr1/1(arm_count) -> Terminator17/1
  fd_tr1/4(ld_count) -> Terminator19/1
  fd_tr1/3(enable) -> [fd_en1]
  cd_tr1/3(enable) -> [cd_en1]
  cd_tr1/4(ld_count) -> Convert5/1
  delay_tr_status2/1 -> Terminator18/1
  cd_tr2/1(arm_count) -> Convert2/1
  cd_tr2/4(ld_count) -> Convert3/1
  cd_tr2/3(enable) -> [cd_en2]
  cd_tr2/2(value) -> [cd_val2]
  Convert2/1 -> Concat11/1
  Convert3/1 -> Concat11/2
  Concat11/1 -> delay_tr_status2/1
  fd_tr2/2(value) -> [fd_val2]
  fd_tr2/3(enable) -> [fd_en2]
  delay_tr_status3/1 -> Terminator21/1
  cd_tr3/1(arm_count) -> Convert6/1
  cd_tr3/2(value) -> [cd_val3]
  Convert6/1 -> Concat4/1
  Convert7/1 -> Concat4/2
  Concat4/1 -> delay_tr_status3/1
  fd_tr3/2(value) -> [fd_val3]
  fd_tr2/1(arm_count) -> Terminator20/1
  fd_tr2/4(ld_count) -> Terminator54/1
  fd_tr3/1(arm_count) -> Terminator52/1
  fd_tr3/4(ld_count) -> Terminator53/1
  fd_tr3/3(enable) -> [fd_en3]
  cd_tr3/3(enable) -> [cd_en3]
  cd_tr3/4(ld_count) -> Convert7/1
  [mrst] -> cd_tr2/1(rst)
  [fd_local_time] -> fd_tr2/3(current_time)
  [mrst] -> cd_tr3/1(rst)
  [cd_local_time] -> cd_tr3/3(current_time)
  [mrst] -> fd_tr3/1(rst)
  [fd_local_time] -> fd_tr3/3(current_time)
  [sync] -> delay_gen0/1(sync_in)
  [cd_en0] -> delay_gen0/2(en)
  Slice50/1 -> delay_gen0/3(phase)
  Slice53/1 -> delay_gen0/4(d/dt_phase)
  [data_adc0] -> delay_gen0/7(din0)
  Constant39/1 -> delay_gen0/8(din1)
  [fd_en0] -> fd_fs0/2(en)
  pol_pack0/1(Out1) -> [adc0]
  pol_pack1/1(Out1) -> [adc1]
  pol_pack2/1(Out1) -> [adc2]
  pol_pack3/1(Out1) -> [adc3]
  coarse_delay0/1 -> Slice38/1
  Slice2/1 -> Concat22/1
  Slice3/1 -> Concat22/2
  Slice38/1 -> Concat22/3
  Concat22/1 -> cd_tr0/5(load_value), fd_tr0/5(load_value)
  Constant12/1 -> ld_time_msw1/1
  Constant10/1 -> ld_time_lsw1/1
  ld_time_msw1/1 -> Slice13/1, Slice39/1
  Slice39/1 -> Concat6/1
  ld_time_lsw1/1 -> Concat6/2
  Constant11/1 -> coarse_delay1/1
  Constant14/1 -> a0_fd1/1
  Constant13/1 -> a1_fd1/1
  a0_fd1/1 -> Slice8/1, Slice9/1
  a1_fd1/1 -> Slice11/1, Slice12/1
  Slice11/1 -> Concat1/4
  Slice12/1 -> Concat1/5
  Slice13/1 -> cd_tr1/2(arm), fd_tr1/2(arm)
  coarse_delay1/1 -> Slice10/1
  Slice8/1 -> Concat1/1
  Slice9/1 -> Concat1/2
  Slice10/1 -> Concat1/3
  Concat6/1 -> cd_tr1/4(load_time), fd_tr1/4(load_time)
  Concat1/1 -> cd_tr1/5(load_value), fd_tr1/5(load_value)
  Constant24/1 -> ld_time_msw2/1
  Constant22/1 -> ld_time_lsw2/1
  ld_time_msw2/1 -> Slice22/1, Slice21/1
  Slice22/1 -> Concat13/1
  ld_time_lsw2/1 -> Concat13/2
  Constant23/1 -> coarse_delay2/1
  Constant26/1 -> a0_fd2/1
  Constant25/1 -> a1_fd2/1
  a0_fd2/1 -> Slice40/1, Slice23/1
  a1_fd2/1 -> Slice20/1, Slice19/1
  Slice19/1 -> Concat12/4
  Slice20/1 -> Concat12/5
  Slice21/1 -> fd_tr2/2(arm), cd_tr2/2(arm)
  coarse_delay2/1 -> Slice18/1
  Slice23/1 -> Concat12/1
  Slice40/1 -> Concat12/2
  Slice18/1 -> Concat12/3
  Concat13/1 -> cd_tr2/4(load_time), fd_tr2/4(load_time)
  Concat12/1 -> fd_tr2/5(load_value), cd_tr2/5(load_value)
  Constant17/1 -> ld_time_msw3/1
  Constant15/1 -> ld_time_lsw3/1
  ld_time_msw3/1 -> Slice17/1, Slice24/1
  Slice24/1 -> Concat15/1
  ld_time_lsw3/1 -> Concat15/2
  Constant16/1 -> coarse_delay3/1
  Constant21/1 -> a0_fd3/1
  Constant20/1 -> a1_fd3/1
  a0_fd3/1 -> Slice25/1, Slice87/1
  a1_fd3/1 -> Slice15/1, Slice16/1
  Slice15/1 -> Concat14/4
  Slice16/1 -> Concat14/5
  Slice17/1 -> cd_tr3/2(arm), fd_tr3/2(arm)
  coarse_delay3/1 -> Slice14/1
  Slice25/1 -> Concat14/1
  Slice87/1 -> Concat14/2
  Slice14/1 -> Concat14/3
  Concat15/1 -> fd_tr3/4(load_time), cd_tr3/4(load_time)
  Concat14/1 -> cd_tr3/5(load_value), fd_tr3/5(load_value)
  Slice52/1 -> Convert9/1
  Slice51/1 -> Concat16/2
  Convert9/1 -> Concat16/1
  Concat16/1 -> delay_gen0/5(delay)
  Slice49/1 -> delay_gen0/6(d/dt_delay)
  Constant53/1 -> delay_gen0/9(invert_slope)
  [sync] -> delay_gen1/1(sync_in)
  [cd_en1] -> delay_gen1/2(en)
  Slice55/1 -> delay_gen1/3(phase)
  Slice75/1 -> delay_gen1/4(d/dt_phase)
  Constant54/1 -> delay_gen1/8(din1)
  Slice74/1 -> Convert8/1
  Slice68/1 -> Concat17/2
  Convert8/1 -> Concat17/1
  Concat17/1 -> delay_gen1/5(delay)
  Slice54/1 -> delay_gen1/6(d/dt_delay)
  Constant55/1 -> delay_gen1/9(invert_slope)
  [cd_val1] -> Slice55/1, Slice54/1, Slice68/1, Slice74/1, Slice75/1
  delay_gen2/4(phase_out) -> Terminator45/1
  delay_gen2/5(slope_out) -> Terminator46/1
  delay_gen2/9(idx_d1) -> Terminator49/1
  delay_gen2/8(d1) -> Terminator48/1
  delay_gen2/7(idx_d0) -> Terminator47/1
  delay_gen2/6(d0) -> unpack2/1(In1)
  [sync] -> delay_gen2/1(sync_in)
  [cd_en2] -> delay_gen2/2(en)
  Slice77/1 -> delay_gen2/3(phase)
  Slice81/1 -> delay_gen2/4(d/dt_phase)
  Constant47/1 -> delay_gen2/8(din1)
  Slice80/1 -> Convert10/1
  Slice79/1 -> Concat18/2
  Convert10/1 -> Concat18/1
  Concat18/1 -> delay_gen2/5(delay)
  Slice76/1 -> delay_gen2/6(d/dt_delay)
  Constant51/1 -> delay_gen2/9(invert_slope)
  [cd_val2] -> Slice81/1, Slice80/1, Slice79/1, Slice76/1, Slice77/1
  [data_adc2] -> delay_gen2/7(din0)
  delay_gen2/1(sync_out) -> delay_wideband_prog2/1(sync)
  unpack2/1(Out1) -> delay_wideband_prog2/4(data_in1)
  unpack2/2(Out2) -> delay_wideband_prog2/5(data_in2)
  unpack2/3(Out3) -> delay_wideband_prog2/6(data_in3)
  unpack2/4(Out4) -> delay_wideband_prog2/7(data_in4)
  delay_gen3/4(phase_out) -> Terminator50/1
  delay_gen3/5(slope_out) -> Terminator55/1
  delay_gen3/9(idx_d1) -> Terminator58/1
  delay_gen3/8(d1) -> Terminator57/1
  delay_gen3/7(idx_d0) -> Terminator56/1
  delay_gen3/6(d0) -> unpack3/1(In1)
  [sync] -> delay_gen3/1(sync_in)
  [cd_en3] -> delay_gen3/2(en)
  Slice83/1 -> delay_gen3/3(phase)
  Slice86/1 -> delay_gen3/4(d/dt_phase)
  Constant52/1 -> delay_gen3/8(din1)
  Slice85/1 -> Convert11/1
  Slice84/1 -> Concat19/2
  Convert11/1 -> Concat19/1
  Concat19/1 -> delay_gen3/5(delay)
  Slice82/1 -> delay_gen3/6(d/dt_delay)
  Constant56/1 -> delay_gen3/9(invert_slope)
  [cd_val3] -> Slice83/1, Slice82/1, Slice84/1, Slice85/1, Slice86/1
  [data_adc2] -> delay_gen3/7(din0)
  delay_gen3/1(sync_out) -> delay_wideband_prog3/1(sync)
  delay_gen3/2(en_out) -> delay_wideband_prog3/3(en)
  delay_gen3/3(cd_o) -> delay_wideband_prog3/2(delay)
  unpack3/1(Out1) -> delay_wideband_prog3/4(data_in1)
  unpack3/2(Out2) -> delay_wideband_prog3/5(data_in2)
  unpack3/3(Out3) -> delay_wideband_prog3/6(data_in3)
  unpack3/4(Out4) -> delay_wideband_prog3/7(data_in4)
  Slice88/1 -> fd_fs0/4(d/dt_phase)
  Slice69/1 -> fd_fs0/5(cd)
  Slice57/1 -> fd_fs0/3(phase)
  Slice58/1 -> fd_fs0/6(frac_delay)
  Slice56/1 -> fd_fs0/7(d/dt_delay)
  [fd_val0] -> Slice57/1, Slice56/1, Slice58/1, Slice69/1, Slice88/1
  [fd_en1] -> fd_fs1/2(en)
  Slice89/1 -> fd_fs1/4(d/dt_phase)
  Slice62/1 -> fd_fs1/5(cd)
  Slice60/1 -> fd_fs1/3(phase)
  Slice61/1 -> fd_fs1/6(frac_delay)
  Slice59/1 -> fd_fs1/7(d/dt_delay)
  [fd_val1] -> Slice89/1, Slice62/1, Slice61/1, Slice59/1, Slice60/1
  fft_wideband_real1/1(sync_out) -> fd_fs1/1(sync)
  fft_wideband_real1/2(out0) -> fd_fs1/8(din0)
  fft_wideband_real1/3(out1) -> fd_fs1/9(din1)
  [fd_en2] -> fd_fs2/2(en)
  Slice67/1 -> fd_fs2/4(d/dt_phase)
  Slice66/1 -> fd_fs2/5(cd)
  Slice64/1 -> fd_fs2/3(phase)
  Slice65/1 -> fd_fs2/6(frac_delay)
  Slice63/1 -> fd_fs2/7(d/dt_delay)
  [fd_val2] -> Slice64/1, Slice63/1, Slice65/1, Slice66/1, Slice67/1
  fft_wideband_real2/1(sync_out) -> fd_fs2/1(sync)
  fft_wideband_real2/2(out0) -> fd_fs2/8(din0)
  fft_wideband_real2/3(out1) -> fd_fs2/9(din1)
  [fd_val3] -> Slice91/1, Slice90/1, Slice72/1, Slice70/1, Slice71/1
  delay_gen0/1(sync_out) -> Gateway Out11/1, delay_wideband_prog0/1(sync)
  delay_gen0/2(en_out) -> delay_wideband_prog0/3(en)
  delay_gen0/3(cd_o) -> Gateway Out16/1, delay_wideband_prog0/2(delay)
  delay_gen0/4(phase_out) -> Terminator35/1
  delay_gen0/5(slope_out) -> Terminator36/1
  delay_gen0/7(idx_d0) -> Terminator37/1
  delay_gen0/8(d1) -> Terminator38/1
  delay_gen0/9(idx_d1) -> Terminator39/1
  delay_gen1/9(idx_d1) -> Terminator44/1
  delay_gen1/8(d1) -> Terminator43/1
  delay_gen1/7(idx_d0) -> Terminator42/1
  delay_gen1/1(sync_out) -> delay_wideband_prog1/1(sync)
  delay_gen1/2(en_out) -> delay_wideband_prog1/3(en)
  delay_gen1/3(cd_o) -> delay_wideband_prog1/2(delay)
  delay_gen1/4(phase_out) -> Terminator40/1
  delay_gen1/5(slope_out) -> Terminator41/1
  delay_gen1/6(d0) -> unpack1/1(In1)
  unpack1/1(Out1) -> delay_wideband_prog1/4(data_in1)
  unpack1/2(Out2) -> delay_wideband_prog1/5(data_in2)
  unpack1/3(Out3) -> delay_wideband_prog1/6(data_in3)
  unpack1/4(Out4) -> delay_wideband_prog1/7(data_in4)
  delay_gen2/3(cd_o) -> delay_wideband_prog2/2(delay)
  delay_gen2/2(en_out) -> delay_wideband_prog2/3(en)
  delay_gen0/6(d0) -> unpack0/1(In1)
  [data_adc1] -> delay_gen1/7(din0)
  [fd_en3] -> fd_fs3/2(en)
  fft_wideband_real3/1(sync_out) -> fd_fs3/1(sync)
  Slice71/1 -> fd_fs3/3(phase)
  Slice91/1 -> fd_fs3/4(d/dt_phase)
  Slice90/1 -> fd_fs3/5(cd)
  Slice72/1 -> fd_fs3/6(frac_delay)
  Slice70/1 -> fd_fs3/7(d/dt_delay)
  fft_wideband_real3/2(out0) -> fd_fs3/8(din0)
  fft_wideband_real3/3(out1) -> fd_fs3/9(din1)
